simulink model "f0"  (Simulink 8.0) (continued)
== system f0/FIR/FIR_prog == (continued)
      mask help: A delay block that uses BRAM for its storage.
      mask init:
      | delay_bram_init(gcb, ...
      |     'DelayLen', DelayLen, ...
      |     'bram_latency', bram_latency, ...
      |     'use_dsp48', use_dsp48, ...
      |     'async', async);
      mask Help: eval('xlWeb(''http://casper.berkeley.edu/wiki/Delay_bram'')')
  delay6 : Subsystem  AncestorBlock=casper_library_delays/delay_bram  mask(delay_bram){DelayLen=256, bram_latency=2, use_dsp48=off, async=off}
      mask prompts: DelayLen "Delay By:"; bram_latency "BRAM Latency"; use_dsp48 "Use DSP48"; async "asynchronous operation"
      mask help: A delay block that uses BRAM for its storage.
      mask init:
      | delay_bram_init(gcb, ...
      |     'DelayLen', DelayLen, ...
      |     'bram_latency', bram_latency, ...
      |     'use_dsp48', use_dsp48, ...
      |     'async', async);
      mask Help: eval('xlWeb(''http://casper.berkeley.edu/wiki/Delay_bram'')')
  delay60 : Subsystem  AncestorBlock=casper_library_delays/delay_bram  mask(delay_bram){DelayLen=256, bram_latency=2, use_dsp48=off, async=off}
      mask prompts: DelayLen "Delay By:"; bram_latency "BRAM Latency"; use_dsp48 "Use DSP48"; async "asynchronous operation"
      mask help: A delay block that uses BRAM for its storage.
      mask init:
      | delay_bram_init(gcb, ...
      |     'DelayLen', DelayLen, ...
      |     'bram_latency', bram_latency, ...
      |     'use_dsp48', use_dsp48, ...
      |     'async', async);
      mask Help: eval('xlWeb(''http://casper.berkeley.edu/wiki/Delay_bram'')')
  delay61 : Subsystem  AncestorBlock=casper_library_delays/delay_bram  mask(delay_bram){DelayLen=256, bram_latency=2, use_dsp48=off, async=off}
      mask prompts: DelayLen "Delay By:"; bram_latency "BRAM Latency"; use_dsp48 "Use DSP48"; async "asynchronous operation"
      mask help: A delay block that uses BRAM for its storage.
      mask init:
      | delay_bram_init(gcb, ...
      |     'DelayLen', DelayLen, ...
      |     'bram_latency', bram_latency, ...
      |     'use_dsp48', use_dsp48, ...
      |     'async', async);
      mask Help: eval('xlWeb(''http://casper.berkeley.edu/wiki/Delay_bram'')')
  delay62 : Subsystem  AncestorBlock=casper_library_delays/delay_bram  mask(delay_bram){DelayLen=256, bram_latency=2, use_dsp48=off, async=off}
      mask prompts: DelayLen "Delay By:"; bram_latency "BRAM Latency"; use_dsp48 "Use DSP48"; async "asynchronous operation"
      mask help: A delay block that uses BRAM for its storage.
      mask init:
      | delay_bram_init(gcb, ...
      |     'DelayLen', DelayLen, ...
      |     'bram_latency', bram_latency, ...
      |     'use_dsp48', use_dsp48, ...
      |     'async', async);
      mask Help: eval('xlWeb(''http://casper.berkeley.edu/wiki/Delay_bram'')')
  delay63 : Subsystem  AncestorBlock=casper_library_delays/delay_bram  mask(delay_bram){DelayLen=256, bram_latency=2, use_dsp48=off, async=off}
      mask prompts: DelayLen "Delay By:"; bram_latency "BRAM Latency"; use_dsp48 "Use DSP48"; async "asynchronous operation"
      mask help: A delay block that uses BRAM for its storage.
      mask init:
      | delay_bram_init(gcb, ...
      |     'DelayLen', DelayLen, ...
      |     'bram_latency', bram_latency, ...
      |     'use_dsp48', use_dsp48, ...
      |     'async', async);
      mask Help: eval('xlWeb(''http://casper.berkeley.edu/wiki/Delay_bram'')')
  delay64 : Subsystem  AncestorBlock=casper_library_delays/delay_bram  mask(delay_bram){DelayLen=256, bram_latency=2, use_dsp48=off, async=off}
      mask prompts: DelayLen "Delay By:"; bram_latency "BRAM Latency"; use_dsp48 "Use DSP48"; async "asynchronous operation"
      mask help: A delay block that uses BRAM for its storage.
      mask init:
      | delay_bram_init(gcb, ...
      |     'DelayLen', DelayLen, ...
      |     'bram_latency', bram_latency, ...
      |     'use_dsp48', use_dsp48, ...
      |     'async', async);
      mask Help: eval('xlWeb(''http://casper.berkeley.edu/wiki/Delay_bram'')')
  delay65 : Subsystem  AncestorBlock=casper_library_delays/delay_bram  mask(delay_bram){DelayLen=256, bram_latency=2, use_dsp48=off, async=off}
      mask prompts: DelayLen "Delay By:"; bram_latency "BRAM Latency"; use_dsp48 "Use DSP48"; async "asynchronous operation"
      mask help: A delay block that uses BRAM for its storage.
      mask init:
      | delay_bram_init(gcb, ...
      |     'DelayLen', DelayLen, ...
      |     'bram_latency', bram_latency, ...
      |     'use_dsp48', use_dsp48, ...
      |     'async', async);
      mask Help: eval('xlWeb(''http://casper.berkeley.edu/wiki/Delay_bram'')')
  delay66 : Subsystem  AncestorBlock=casper_library_delays/delay_bram  mask(delay_bram){DelayLen=256, bram_latency=2, use_dsp48=off, async=off}
      mask prompts: DelayLen "Delay By:"; bram_latency "BRAM Latency"; use_dsp48 "Use DSP48"; async "asynchronous operation"
      mask help: A delay block that uses BRAM for its storage.
      mask init:
      | delay_bram_init(gcb, ...
      |     'DelayLen', DelayLen, ...
      |     'bram_latency', bram_latency, ...
      |     'use_dsp48', use_dsp48, ...
      |     'async', async);
      mask Help: eval('xlWeb(''http://casper.berkeley.edu/wiki/Delay_bram'')')
  delay67 : Subsystem  AncestorBlock=casper_library_delays/delay_bram  mask(delay_bram){DelayLen=256, bram_latency=2, use_dsp48=off, async=off}
      mask prompts: DelayLen "Delay By:"; bram_latency "BRAM Latency"; use_dsp48 "Use DSP48"; async "asynchronous operation"
      mask help: A delay block that uses BRAM for its storage.
      mask init:
      | delay_bram_init(gcb, ...
      |     'DelayLen', DelayLen, ...
      |     'bram_latency', bram_latency, ...
      |     'use_dsp48', use_dsp48, ...
      |     'async', async);
      mask Help: eval('xlWeb(''http://casper.berkeley.edu/wiki/Delay_bram'')')
  delay68 : Subsystem  AncestorBlock=casper_library_delays/delay_bram  mask(delay_bram){DelayLen=256, bram_latency=2, use_dsp48=off, async=off}
      mask prompts: DelayLen "Delay By:"; bram_latency "BRAM Latency"; use_dsp48 "Use DSP48"; async "asynchronous operation"
      mask help: A delay block that uses BRAM for its storage.
      mask init:
      | delay_bram_init(gcb, ...
      |     'DelayLen', DelayLen, ...
      |     'bram_latency', bram_latency, ...
      |     'use_dsp48', use_dsp48, ...
      |     'async', async);
      mask Help: eval('xlWeb(''http://casper.berkeley.edu/wiki/Delay_bram'')')
  delay69 : Subsystem  AncestorBlock=casper_library_delays/delay_bram  mask(delay_bram){DelayLen=256, bram_latency=2, use_dsp48=off, async=off}
      mask prompts: DelayLen "Delay By:"; bram_latency "BRAM Latency"; use_dsp48 "Use DSP48"; async "asynchronous operation"
      mask help: A delay block that uses BRAM for its storage.
      mask init:
      | delay_bram_init(gcb, ...
      |     'DelayLen', DelayLen, ...
      |     'bram_latency', bram_latency, ...
      |     'use_dsp48', use_dsp48, ...
      |     'async', async);
      mask Help: eval('xlWeb(''http://casper.berkeley.edu/wiki/Delay_bram'')')
  delay7 : Subsystem  AncestorBlock=casper_library_delays/delay_bram  mask(delay_bram){DelayLen=256, bram_latency=2, use_dsp48=off, async=off}
      mask prompts: DelayLen "Delay By:"; bram_latency "BRAM Latency"; use_dsp48 "Use DSP48"; async "asynchronous operation"
      mask help: A delay block that uses BRAM for its storage.
      mask init:
      | delay_bram_init(gcb, ...
      |     'DelayLen', DelayLen, ...
      |     'bram_latency', bram_latency, ...
      |     'use_dsp48', use_dsp48, ...
      |     'async', async);
      mask Help: eval('xlWeb(''http://casper.berkeley.edu/wiki/Delay_bram'')')
  delay70 : Subsystem  AncestorBlock=casper_library_delays/delay_bram  mask(delay_bram){DelayLen=256, bram_latency=2, use_dsp48=off, async=off}
      mask prompts: DelayLen "Delay By:"; bram_latency "BRAM Latency"; use_dsp48 "Use DSP48"; async "asynchronous operation"
      mask help: A delay block that uses BRAM for its storage.
      mask init:
      | delay_bram_init(gcb, ...
      |     'DelayLen', DelayLen, ...
      |     'bram_latency', bram_latency, ...
      |     'use_dsp48', use_dsp48, ...
      |     'async', async);
      mask Help: eval('xlWeb(''http://casper.berkeley.edu/wiki/Delay_bram'')')
  delay71 : Subsystem  AncestorBlock=casper_library_delays/delay_bram  mask(delay_bram){DelayLen=256, bram_latency=2, use_dsp48=off, async=off}
      mask prompts: DelayLen "Delay By:"; bram_latency "BRAM Latency"; use_dsp48 "Use DSP48"; async "asynchronous operation"
      mask help: A delay block that uses BRAM for its storage.
      mask init:
      | delay_bram_init(gcb, ...
      |     'DelayLen', DelayLen, ...
      |     'bram_latency', bram_latency, ...
      |     'use_dsp48', use_dsp48, ...
      |     'async', async);
      mask Help: eval('xlWeb(''http://casper.berkeley.edu/wiki/Delay_bram'')')
  delay72 : Subsystem  AncestorBlock=casper_library_delays/delay_bram  mask(delay_bram){DelayLen=256, bram_latency=2, use_dsp48=off, async=off}
      mask prompts: DelayLen "Delay By:"; bram_latency "BRAM Latency"; use_dsp48 "Use DSP48"; async "asynchronous operation"
      mask help: A delay block that uses BRAM for its storage.
      mask init:
      | delay_bram_init(gcb, ...
      |     'DelayLen', DelayLen, ...
      |     'bram_latency', bram_latency, ...
      |     'use_dsp48', use_dsp48, ...
      |     'async', async);
      mask Help: eval('xlWeb(''http://casper.berkeley.edu/wiki/Delay_bram'')')
  delay73 : Subsystem  AncestorBlock=casper_library_delays/delay_bram  mask(delay_bram){DelayLen=256, bram_latency=2, use_dsp48=off, async=off}
      mask prompts: DelayLen "Delay By:"; bram_latency "BRAM Latency"; use_dsp48 "Use DSP48"; async "asynchronous operation"
      mask help: A delay block that uses BRAM for its storage.
      mask init:
      | delay_bram_init(gcb, ...
      |     'DelayLen', DelayLen, ...
      |     'bram_latency', bram_latency, ...
      |     'use_dsp48', use_dsp48, ...
      |     'async', async);
      mask Help: eval('xlWeb(''http://casper.berkeley.edu/wiki/Delay_bram'')')
  delay74 : Subsystem  AncestorBlock=casper_library_delays/delay_bram  mask(delay_bram){DelayLen=256, bram_latency=2, use_dsp48=off, async=off}
      mask prompts: DelayLen "Delay By:"; bram_latency "BRAM Latency"; use_dsp48 "Use DSP48"; async "asynchronous operation"
      mask help: A delay block that uses BRAM for its storage.
      mask init:
      | delay_bram_init(gcb, ...
      |     'DelayLen', DelayLen, ...
      |     'bram_latency', bram_latency, ...
      |     'use_dsp48', use_dsp48, ...
      |     'async', async);
      mask Help: eval('xlWeb(''http://casper.berkeley.edu/wiki/Delay_bram'')')
  delay75 : Subsystem  AncestorBlock=casper_library_delays/delay_bram  mask(delay_bram){DelayLen=256, bram_latency=2, use_dsp48=off, async=off}
      mask prompts: DelayLen "Delay By:"; bram_latency "BRAM Latency"; use_dsp48 "Use DSP48"; async "asynchronous operation"
      mask help: A delay block that uses BRAM for its storage.
      mask init:
      | delay_bram_init(gcb, ...
      |     'DelayLen', DelayLen, ...
      |     'bram_latency', bram_latency, ...
      |     'use_dsp48', use_dsp48, ...
      |     'async', async);
      mask Help: eval('xlWeb(''http://casper.berkeley.edu/wiki/Delay_bram'')')
  delay76 : Subsystem  AncestorBlock=casper_library_delays/delay_bram  mask(delay_bram){DelayLen=256, bram_latency=2, use_dsp48=off, async=off}
      mask prompts: DelayLen "Delay By:"; bram_latency "BRAM Latency"; use_dsp48 "Use DSP48"; async "asynchronous operation"
      mask help: A delay block that uses BRAM for its storage.
      mask init:
      | delay_bram_init(gcb, ...
      |     'DelayLen', DelayLen, ...
      |     'bram_latency', bram_latency, ...
      |     'use_dsp48', use_dsp48, ...
      |     'async', async);
      mask Help: eval('xlWeb(''http://casper.berkeley.edu/wiki/Delay_bram'')')
  delay77 : Subsystem  AncestorBlock=casper_library_delays/delay_bram  mask(delay_bram){DelayLen=256, bram_latency=2, use_dsp48=off, async=off}
      mask prompts: DelayLen "Delay By:"; bram_latency "BRAM Latency"; use_dsp48 "Use DSP48"; async "asynchronous operation"
      mask help: A delay block that uses BRAM for its storage.
      mask init:
      | delay_bram_init(gcb, ...
      |     'DelayLen', DelayLen, ...
      |     'bram_latency', bram_latency, ...
      |     'use_dsp48', use_dsp48, ...
      |     'async', async);
      mask Help: eval('xlWeb(''http://casper.berkeley.edu/wiki/Delay_bram'')')
  delay78 : Subsystem  AncestorBlock=casper_library_delays/delay_bram  mask(delay_bram){DelayLen=256, bram_latency=2, use_dsp48=off, async=off}
      mask prompts: DelayLen "Delay By:"; bram_latency "BRAM Latency"; use_dsp48 "Use DSP48"; async "asynchronous operation"
      mask help: A delay block that uses BRAM for its storage.
      mask init:
      | delay_bram_init(gcb, ...
      |     'DelayLen', DelayLen, ...
      |     'bram_latency', bram_latency, ...
      |     'use_dsp48', use_dsp48, ...
      |     'async', async);
      mask Help: eval('xlWeb(''http://casper.berkeley.edu/wiki/Delay_bram'')')
  delay79 : Subsystem  AncestorBlock=casper_library_delays/delay_bram  mask(delay_bram){DelayLen=256, bram_latency=2, use_dsp48=off, async=off}
      mask prompts: DelayLen "Delay By:"; bram_latency "BRAM Latency"; use_dsp48 "Use DSP48"; async "asynchronous operation"
      mask help: A delay block that uses BRAM for its storage.
      mask init:
      | delay_bram_init(gcb, ...
      |     'DelayLen', DelayLen, ...
      |     'bram_latency', bram_latency, ...
      |     'use_dsp48', use_dsp48, ...
      |     'async', async);
      mask Help: eval('xlWeb(''http://casper.berkeley.edu/wiki/Delay_bram'')')
  delay8 : Subsystem  AncestorBlock=casper_library_delays/delay_bram  mask(delay_bram){DelayLen=256, bram_latency=2, use_dsp48=off, async=off}
      mask prompts: DelayLen "Delay By:"; bram_latency "BRAM Latency"; use_dsp48 "Use DSP48"; async "asynchronous operation"
      mask help: A delay block that uses BRAM for its storage.
      mask init:
      | delay_bram_init(gcb, ...
      |     'DelayLen', DelayLen, ...
      |     'bram_latency', bram_latency, ...
      |     'use_dsp48', use_dsp48, ...
      |     'async', async);
      mask Help: eval('xlWeb(''http://casper.berkeley.edu/wiki/Delay_bram'')')
  delay80 : Subsystem  AncestorBlock=casper_library_delays/delay_bram  mask(delay_bram){DelayLen=256, bram_latency=2, use_dsp48=off, async=off}
      mask prompts: DelayLen "Delay By:"; bram_latency "BRAM Latency"; use_dsp48 "Use DSP48"; async "asynchronous operation"
      mask help: A delay block that uses BRAM for its storage.
      mask init:
      | delay_bram_init(gcb, ...
      |     'DelayLen', DelayLen, ...
      |     'bram_latency', bram_latency, ...
      |     'use_dsp48', use_dsp48, ...
      |     'async', async);
      mask Help: eval('xlWeb(''http://casper.berkeley.edu/wiki/Delay_bram'')')
  delay81 : Subsystem  AncestorBlock=casper_library_delays/delay_bram  mask(delay_bram){DelayLen=256, bram_latency=2, use_dsp48=off, async=off}
      mask prompts: DelayLen "Delay By:"; bram_latency "BRAM Latency"; use_dsp48 "Use DSP48"; async "asynchronous operation"
      mask help: A delay block that uses BRAM for its storage.
      mask init:
      | delay_bram_init(gcb, ...
      |     'DelayLen', DelayLen, ...
      |     'bram_latency', bram_latency, ...
      |     'use_dsp48', use_dsp48, ...
      |     'async', async);
      mask Help: eval('xlWeb(''http://casper.berkeley.edu/wiki/Delay_bram'')')
  delay82 : Subsystem  AncestorBlock=casper_library_delays/delay_bram  mask(delay_bram){DelayLen=256, bram_latency=2, use_dsp48=off, async=off}
      mask prompts: DelayLen "Delay By:"; bram_latency "BRAM Latency"; use_dsp48 "Use DSP48"; async "asynchronous operation"
      mask help: A delay block that uses BRAM for its storage.
      mask init:
      | delay_bram_init(gcb, ...
      |     'DelayLen', DelayLen, ...
      |     'bram_latency', bram_latency, ...
      |     'use_dsp48', use_dsp48, ...
      |     'async', async);
      mask Help: eval('xlWeb(''http://casper.berkeley.edu/wiki/Delay_bram'')')
  delay83 : Subsystem  AncestorBlock=casper_library_delays/delay_bram  mask(delay_bram){DelayLen=256, bram_latency=2, use_dsp48=off, async=off}
      mask prompts: DelayLen "Delay By:"; bram_latency "BRAM Latency"; use_dsp48 "Use DSP48"; async "asynchronous operation"
      mask help: A delay block that uses BRAM for its storage.
      mask init:
      | delay_bram_init(gcb, ...
      |     'DelayLen', DelayLen, ...
      |     'bram_latency', bram_latency, ...
      |     'use_dsp48', use_dsp48, ...
      |     'async', async);
      mask Help: eval('xlWeb(''http://casper.berkeley.edu/wiki/Delay_bram'')')
  delay84 : Subsystem  AncestorBlock=casper_library_delays/delay_bram  mask(delay_bram){DelayLen=256, bram_latency=2, use_dsp48=off, async=off}
      mask prompts: DelayLen "Delay By:"; bram_latency "BRAM Latency"; use_dsp48 "Use DSP48"; async "asynchronous operation"
      mask help: A delay block that uses BRAM for its storage.
      mask init:
      | delay_bram_init(gcb, ...
      |     'DelayLen', DelayLen, ...
      |     'bram_latency', bram_latency, ...
      |     'use_dsp48', use_dsp48, ...
      |     'async', async);
      mask Help: eval('xlWeb(''http://casper.berkeley.edu/wiki/Delay_bram'')')
  delay85 : Subsystem  AncestorBlock=casper_library_delays/delay_bram  mask(delay_bram){DelayLen=256, bram_latency=2, use_dsp48=off, async=off}
      mask prompts: DelayLen "Delay By:"; bram_latency "BRAM Latency"; use_dsp48 "Use DSP48"; async "asynchronous operation"
      mask help: A delay block that uses BRAM for its storage.
      mask init:
      | delay_bram_init(gcb, ...
      |     'DelayLen', DelayLen, ...
      |     'bram_latency', bram_latency, ...
      |     'use_dsp48', use_dsp48, ...
      |     'async', async);
      mask Help: eval('xlWeb(''http://casper.berkeley.edu/wiki/Delay_bram'')')
  delay86 : Subsystem  AncestorBlock=casper_library_delays/delay_bram  mask(delay_bram){DelayLen=256, bram_latency=2, use_dsp48=off, async=off}
      mask prompts: DelayLen "Delay By:"; bram_latency "BRAM Latency"; use_dsp48 "Use DSP48"; async "asynchronous operation"
      mask help: A delay block that uses BRAM for its storage.
      mask init:
      | delay_bram_init(gcb, ...
      |     'DelayLen', DelayLen, ...
      |     'bram_latency', bram_latency, ...
      |     'use_dsp48', use_dsp48, ...
      |     'async', async);
      mask Help: eval('xlWeb(''http://casper.berkeley.edu/wiki/Delay_bram'')')
  delay87 : Subsystem  AncestorBlock=casper_library_delays/delay_bram  mask(delay_bram){DelayLen=256, bram_latency=2, use_dsp48=off, async=off}
      mask prompts: DelayLen "Delay By:"; bram_latency "BRAM Latency"; use_dsp48 "Use DSP48"; async "asynchronous operation"
      mask help: A delay block that uses BRAM for its storage.
      mask init:
      | delay_bram_init(gcb, ...
      |     'DelayLen', DelayLen, ...
      |     'bram_latency', bram_latency, ...
      |     'use_dsp48', use_dsp48, ...
      |     'async', async);
      mask Help: eval('xlWeb(''http://casper.berkeley.edu/wiki/Delay_bram'')')
  delay88 : Subsystem  AncestorBlock=casper_library_delays/delay_bram  mask(delay_bram){DelayLen=256, bram_latency=2, use_dsp48=off, async=off}
      mask prompts: DelayLen "Delay By:"; bram_latency "BRAM Latency"; use_dsp48 "Use DSP48"; async "asynchronous operation"
      mask help: A delay block that uses BRAM for its storage.
      mask init:
      | delay_bram_init(gcb, ...
      |     'DelayLen', DelayLen, ...
      |     'bram_latency', bram_latency, ...
      |     'use_dsp48', use_dsp48, ...
      |     'async', async);
      mask Help: eval('xlWeb(''http://casper.berkeley.edu/wiki/Delay_bram'')')
  delay89 : Subsystem  AncestorBlock=casper_library_delays/delay_bram  mask(delay_bram){DelayLen=256, bram_latency=2, use_dsp48=off, async=off}
      mask prompts: DelayLen "Delay By:"; bram_latency "BRAM Latency"; use_dsp48 "Use DSP48"; async "asynchronous operation"
      mask help: A delay block that uses BRAM for its storage.
      mask init:
      | delay_bram_init(gcb, ...
      |     'DelayLen', DelayLen, ...
      |     'bram_latency', bram_latency, ...
      |     'use_dsp48', use_dsp48, ...
      |     'async', async);
      mask Help: eval('xlWeb(''http://casper.berkeley.edu/wiki/Delay_bram'')')
  delay9 : Subsystem  AncestorBlock=casper_library_delays/delay_bram  mask(delay_bram){DelayLen=256, bram_latency=2, use_dsp48=off, async=off}
      mask prompts: DelayLen "Delay By:"; bram_latency "BRAM Latency"; use_dsp48 "Use DSP48"; async "asynchronous operation"
      mask help: A delay block that uses BRAM for its storage.
      mask init:
      | delay_bram_init(gcb, ...
      |     'DelayLen', DelayLen, ...
      |     'bram_latency', bram_latency, ...
      |     'use_dsp48', use_dsp48, ...
      |     'async', async);
      mask Help: eval('xlWeb(''http://casper.berkeley.edu/wiki/Delay_bram'')')
  delay90 : Subsystem  AncestorBlock=casper_library_delays/delay_bram  mask(delay_bram){DelayLen=256, bram_latency=2, use_dsp48=off, async=off}
      mask prompts: DelayLen "Delay By:"; bram_latency "BRAM Latency"; use_dsp48 "Use DSP48"; async "asynchronous operation"
      mask help: A delay block that uses BRAM for its storage.
      mask init:
      | delay_bram_init(gcb, ...
      |     'DelayLen', DelayLen, ...
      |     'bram_latency', bram_latency, ...
      |     'use_dsp48', use_dsp48, ...
      |     'async', async);
      mask Help: eval('xlWeb(''http://casper.berkeley.edu/wiki/Delay_bram'')')
  delay91 : Subsystem  AncestorBlock=casper_library_delays/delay_bram  mask(delay_bram){DelayLen=256, bram_latency=2, use_dsp48=off, async=off}
      mask prompts: DelayLen "Delay By:"; bram_latency "BRAM Latency"; use_dsp48 "Use DSP48"; async "asynchronous operation"
      mask help: A delay block that uses BRAM for its storage.
      mask init:
      | delay_bram_init(gcb, ...
      |     'DelayLen', DelayLen, ...
      |     'bram_latency', bram_latency, ...
      |     'use_dsp48', use_dsp48, ...
      |     'async', async);
      mask Help: eval('xlWeb(''http://casper.berkeley.edu/wiki/Delay_bram'')')
  delay92 : Subsystem  AncestorBlock=casper_library_delays/delay_bram  mask(delay_bram){DelayLen=256, bram_latency=2, use_dsp48=off, async=off}
      mask prompts: DelayLen "Delay By:"; bram_latency "BRAM Latency"; use_dsp48 "Use DSP48"; async "asynchronous operation"
      mask help: A delay block that uses BRAM for its storage.
      mask init:
      | delay_bram_init(gcb, ...
      |     'DelayLen', DelayLen, ...
      |     'bram_latency', bram_latency, ...
      |     'use_dsp48', use_dsp48, ...
      |     'async', async);
      mask Help: eval('xlWeb(''http://casper.berkeley.edu/wiki/Delay_bram'')')
  delay93 : Subsystem  AncestorBlock=casper_library_delays/delay_bram  mask(delay_bram){DelayLen=256, bram_latency=2, use_dsp48=off, async=off}
      mask prompts: DelayLen "Delay By:"; bram_latency "BRAM Latency"; use_dsp48 "Use DSP48"; async "asynchronous operation"
      mask help: A delay block that uses BRAM for its storage.
      mask init:
      | delay_bram_init(gcb, ...
      |     'DelayLen', DelayLen, ...
      |     'bram_latency', bram_latency, ...
      |     'use_dsp48', use_dsp48, ...
      |     'async', async);
      mask Help: eval('xlWeb(''http://casper.berkeley.edu/wiki/Delay_bram'')')
  delay94 : Subsystem  AncestorBlock=casper_library_delays/delay_bram  mask(delay_bram){DelayLen=256, bram_latency=2, use_dsp48=off, async=off}
      mask prompts: DelayLen "Delay By:"; bram_latency "BRAM Latency"; use_dsp48 "Use DSP48"; async "asynchronous operation"
      mask help: A delay block that uses BRAM for its storage.
      mask init:
      | delay_bram_init(gcb, ...
      |     'DelayLen', DelayLen, ...
      |     'bram_latency', bram_latency, ...
      |     'use_dsp48', use_dsp48, ...
      |     'async', async);
      mask Help: eval('xlWeb(''http://casper.berkeley.edu/wiki/Delay_bram'')')
  delay95 : Subsystem  AncestorBlock=casper_library_delays/delay_bram  mask(delay_bram){DelayLen=256, bram_latency=2, use_dsp48=off, async=off}
      mask prompts: DelayLen "Delay By:"; bram_latency "BRAM Latency"; use_dsp48 "Use DSP48"; async "asynchronous operation"
      mask help: A delay block that uses BRAM for its storage.
      mask init:
      | delay_bram_init(gcb, ...
      |     'DelayLen', DelayLen, ...
      |     'bram_latency', bram_latency, ...
      |     'use_dsp48', use_dsp48, ...
      |     'async', async);
      mask Help: eval('xlWeb(''http://casper.berkeley.edu/wiki/Delay_bram'')')
  delay96 : Subsystem  AncestorBlock=casper_library_delays/delay_bram  mask(delay_bram){DelayLen=256, bram_latency=2, use_dsp48=off, async=off}
      mask prompts: DelayLen "Delay By:"; bram_latency "BRAM Latency"; use_dsp48 "Use DSP48"; async "asynchronous operation"
      mask help: A delay block that uses BRAM for its storage.
      mask init:
      | delay_bram_init(gcb, ...
      |     'DelayLen', DelayLen, ...
      |     'bram_latency', bram_latency, ...
      |     'use_dsp48', use_dsp48, ...
      |     'async', async);
      mask Help: eval('xlWeb(''http://casper.berkeley.edu/wiki/Delay_bram'')')
  delay97 : Subsystem  AncestorBlock=casper_library_delays/delay_bram  mask(delay_bram){DelayLen=256, bram_latency=2, use_dsp48=off, async=off}
      mask prompts: DelayLen "Delay By:"; bram_latency "BRAM Latency"; use_dsp48 "Use DSP48"; async "asynchronous operation"
      mask help: A delay block that uses BRAM for its storage.
      mask init:
      | delay_bram_init(gcb, ...
      |     'DelayLen', DelayLen, ...
      |     'bram_latency', bram_latency, ...
      |     'use_dsp48', use_dsp48, ...
      |     'async', async);
      mask Help: eval('xlWeb(''http://casper.berkeley.edu/wiki/Delay_bram'')')
  delay98 : Subsystem  AncestorBlock=casper_library_delays/delay_bram  mask(delay_bram){DelayLen=256, bram_latency=2, use_dsp48=off, async=off}
      mask prompts: DelayLen "Delay By:"; bram_latency "BRAM Latency"; use_dsp48 "Use DSP48"; async "asynchronous operation"
      mask help: A delay block that uses BRAM for its storage.
      mask init:
      | delay_bram_init(gcb, ...
      |     'DelayLen', DelayLen, ...
      |     'bram_latency', bram_latency, ...
      |     'use_dsp48', use_dsp48, ...
      |     'async', async);
      mask Help: eval('xlWeb(''http://casper.berkeley.edu/wiki/Delay_bram'')')
  delay99 : Subsystem  AncestorBlock=casper_library_delays/delay_bram  mask(delay_bram){DelayLen=256, bram_latency=2, use_dsp48=off, async=off}
      mask prompts: DelayLen "Delay By:"; bram_latency "BRAM Latency"; use_dsp48 "Use DSP48"; async "asynchronous operation"
      mask help: A delay block that uses BRAM for its storage.
      mask init:
      | delay_bram_init(gcb, ...
      |     'DelayLen', DelayLen, ...
      |     'bram_latency', bram_latency, ...
      |     'use_dsp48', use_dsp48, ...
      |     'async', async);
      mask Help: eval('xlWeb(''http://casper.berkeley.edu/wiki/Delay_bram'')')
  sum : Subsystem  AncestorBlock=casper_library_misc/adder_tree  mask(adder_tree){n_inputs=100, latency=3, adder_imp=DSP48, first_stage_hdl=on}
      mask prompts: n_inputs "Number of Inputs"; latency "Add Latency"; adder_imp "Adder implementation"; first_stage_hdl "Use behavioural HDL for first stage of adders"
      mask help:
      | Sums all inputs using a tree of adds and delays.
      |
      | Number of inputs: The number of inputs to the block to be summed
      | Add latency: The number of clock cycles to perform one addition (affects timing)..
      | Adder implementation: choice of fabric or DSP48E core, or behavioral HDL
      | Use behavioural HDL for first stage of adders: Causes the first stage of adders to be absorbed into DSP blocks if multipliers precede them (Virtex 5). Overrides Adder implementation
      mask init:
      | adder_tree_init(gcb, ...
      |     'n_inputs', n_inputs, ...
      |     'latency', latency, ...
      |     'adder_imp', adder_imp, ...
      |     'first_stage_hdl', first_stage_hdl);
      settings: AttributesFormatString=latency 21
      mask Help: eval('xlWeb(''http://casper.berkeley.edu/wiki/Adder_tree'')')
      mask TypeOptions adder_imp: Behavioral | Fabric | DSP48
  sync_delay : xbsIndex_r4/Delay  rst=off  infoeditControl=Selection of Reset will increase slice count due to use of real FFs and instead of SRLs  en=off  latency=5  dbl_ovrd=off  reg_retiming=off  block_type=delay
  sync_out : Outport
  data_out : Outport  Port=2
  wires:
  sync_in/1 -> sync_delay/1
  sum/2(dout) -> data_out/1
  sync_delay/1 -> sum/1(sync)
  sum/1(sync_out) -> sync_out/1
  coeff1/1 -> Mult1/2
  Mult1/1 -> sum/2(din1)
  coeff2/1 -> Mult2/2
  delay1/1(dout) -> Mult2/1, delay2/1(din)
  Mult2/1 -> sum/3(din2)
  coeff3/1 -> Mult3/2
  delay2/1(dout) -> Mult3/1, delay3/1(din)
  Mult3/1 -> sum/4(din3)
  coeff4/1 -> Mult4/2
  delay3/1(dout) -> Mult4/1, delay4/1(din)
  Mult4/1 -> sum/5(din4)
  coeff5/1 -> Mult5/2
  delay4/1(dout) -> Mult5/1, delay5/1(din)
  Mult5/1 -> sum/6(din5)
  coeff6/1 -> Mult6/2
  delay5/1(dout) -> Mult6/1, delay6/1(din)
  Mult6/1 -> sum/7(din6)
  coeff7/1 -> Mult7/2
  delay6/1(dout) -> Mult7/1, delay7/1(din)
  Mult7/1 -> sum/8(din7)
  coeff8/1 -> Mult8/2
  delay7/1(dout) -> Mult8/1, delay8/1(din)
  Mult8/1 -> sum/9(din8)
  coeff9/1 -> Mult9/2
  delay8/1(dout) -> Mult9/1, delay9/1(din)
  Mult9/1 -> sum/10(din9)
  coeff10/1 -> Mult10/2
  delay9/1(dout) -> Mult10/1, delay10/1(din)
  Mult10/1 -> sum/11(din10)
  coeff11/1 -> Mult11/2
  delay10/1(dout) -> Mult11/1, delay11/1(din)
  Mult11/1 -> sum/12(din11)
  coeff12/1 -> Mult12/2
  delay11/1(dout) -> Mult12/1, delay12/1(din)
  Mult12/1 -> sum/13(din12)
  coeff13/1 -> Mult13/2
  delay12/1(dout) -> Mult13/1, delay13/1(din)
  Mult13/1 -> sum/14(din13)
  coeff14/1 -> Mult14/2
  delay13/1(dout) -> Mult14/1, delay14/1(din)
  Mult14/1 -> sum/15(din14)
  coeff15/1 -> Mult15/2
  delay14/1(dout) -> Mult15/1, delay15/1(din)
  Mult15/1 -> sum/16(din15)
  coeff16/1 -> Mult16/2
  delay15/1(dout) -> Mult16/1, delay16/1(din)
  Mult16/1 -> sum/17(din16)
  coeff17/1 -> Mult17/2
  delay16/1(dout) -> Mult17/1, delay17/1(din)
  Mult17/1 -> sum/18(din17)
  coeff18/1 -> Mult18/2
  delay17/1(dout) -> Mult18/1, delay18/1(din)
  Mult18/1 -> sum/19(din18)
  coeff19/1 -> Mult19/2
  delay18/1(dout) -> Mult19/1, delay19/1(din)
  Mult19/1 -> sum/20(din19)
  coeff20/1 -> Mult20/2
  delay19/1(dout) -> Mult20/1, delay20/1(din)
  Mult20/1 -> sum/21(din20)
  coeff21/1 -> Mult21/2
  delay20/1(dout) -> Mult21/1, delay21/1(din)
  Mult21/1 -> sum/22(din21)
  coeff22/1 -> Mult22/2
  delay21/1(dout) -> Mult22/1, delay22/1(din)
  Mult22/1 -> sum/23(din22)
  coeff23/1 -> Mult23/2
  delay22/1(dout) -> Mult23/1, delay23/1(din)
  Mult23/1 -> sum/24(din23)
  coeff24/1 -> Mult24/2
  delay23/1(dout) -> Mult24/1, delay24/1(din)
  Mult24/1 -> sum/25(din24)
  coeff25/1 -> Mult25/2
  delay24/1(dout) -> Mult25/1, delay25/1(din)
  Mult25/1 -> sum/26(din25)
  coeff26/1 -> Mult26/2
  delay25/1(dout) -> Mult26/1, delay26/1(din)
  Mult26/1 -> sum/27(din26)
  coeff27/1 -> Mult27/2
  delay26/1(dout) -> Mult27/1, delay27/1(din)
  Mult27/1 -> sum/28(din27)
  coeff28/1 -> Mult28/2
  delay27/1(dout) -> Mult28/1, delay28/1(din)
  Mult28/1 -> sum/29(din28)
  coeff29/1 -> Mult29/2
  delay28/1(dout) -> Mult29/1, delay29/1(din)
  Mult29/1 -> sum/30(din29)
  coeff30/1 -> Mult30/2
  delay29/1(dout) -> Mult30/1, delay30/1(din)
  Mult30/1 -> sum/31(din30)
  coeff31/1 -> Mult31/2
  delay30/1(dout) -> Mult31/1, delay31/1(din)
  Mult31/1 -> sum/32(din31)
  coeff32/1 -> Mult32/2
  delay31/1(dout) -> Mult32/1, delay32/1(din)
  Mult32/1 -> sum/33(din32)
  coeff33/1 -> Mult33/2
  delay32/1(dout) -> Mult33/1, delay33/1(din)
  Mult33/1 -> sum/34(din33)
  coeff34/1 -> Mult34/2
  delay33/1(dout) -> Mult34/1, delay34/1(din)
  Mult34/1 -> sum/35(din34)
  coeff35/1 -> Mult35/2
  delay34/1(dout) -> Mult35/1, delay35/1(din)
  Mult35/1 -> sum/36(din35)
  coeff36/1 -> Mult36/2
  delay35/1(dout) -> Mult36/1, delay36/1(din)
  Mult36/1 -> sum/37(din36)
  coeff37/1 -> Mult37/2
  delay36/1(dout) -> Mult37/1, delay37/1(din)
  Mult37/1 -> sum/38(din37)
  coeff38/1 -> Mult38/2
  delay37/1(dout) -> Mult38/1, delay38/1(din)
  Mult38/1 -> sum/39(din38)
  coeff39/1 -> Mult39/2
  delay38/1(dout) -> Mult39/1, delay39/1(din)
  Mult39/1 -> sum/40(din39)
  coeff40/1 -> Mult40/2
  delay39/1(dout) -> Mult40/1, delay40/1(din)
  Mult40/1 -> sum/41(din40)
  coeff41/1 -> Mult41/2
  delay40/1(dout) -> Mult41/1, delay41/1(din)
  Mult41/1 -> sum/42(din41)
  coeff42/1 -> Mult42/2
  delay41/1(dout) -> Mult42/1, delay42/1(din)
  Mult42/1 -> sum/43(din42)
  coeff43/1 -> Mult43/2
  delay42/1(dout) -> Mult43/1, delay43/1(din)
  Mult43/1 -> sum/44(din43)
  coeff44/1 -> Mult44/2
  delay43/1(dout) -> Mult44/1, delay44/1(din)
  Mult44/1 -> sum/45(din44)
  coeff45/1 -> Mult45/2
  delay44/1(dout) -> Mult45/1, delay45/1(din)
  Mult45/1 -> sum/46(din45)
  coeff46/1 -> Mult46/2
  delay45/1(dout) -> Mult46/1, delay46/1(din)
  Mult46/1 -> sum/47(din46)
  coeff47/1 -> Mult47/2
  delay46/1(dout) -> Mult47/1, delay47/1(din)
  Mult47/1 -> sum/48(din47)
  coeff48/1 -> Mult48/2
  delay47/1(dout) -> Mult48/1, delay48/1(din)
  Mult48/1 -> sum/49(din48)
  coeff49/1 -> Mult49/2
  delay48/1(dout) -> Mult49/1, delay49/1(din)
  Mult49/1 -> sum/50(din49)
  coeff50/1 -> Mult50/2
  delay49/1(dout) -> Mult50/1, delay50/1(din)
  Mult50/1 -> sum/51(din50)
  coeff51/1 -> Mult51/2
  delay50/1(dout) -> Mult51/1, delay51/1(din)
  Mult51/1 -> sum/52(din51)
  coeff52/1 -> Mult52/2
  delay51/1(dout) -> Mult52/1, delay52/1(din)
  Mult52/1 -> sum/53(din52)
  coeff53/1 -> Mult53/2
  delay52/1(dout) -> Mult53/1, delay53/1(din)
  Mult53/1 -> sum/54(din53)
  coeff54/1 -> Mult54/2
  delay53/1(dout) -> Mult54/1, delay54/1(din)
  Mult54/1 -> sum/55(din54)
  coeff55/1 -> Mult55/2
  delay54/1(dout) -> Mult55/1, delay55/1(din)
  Mult55/1 -> sum/56(din55)
  coeff56/1 -> Mult56/2
  delay55/1(dout) -> Mult56/1, delay56/1(din)
  Mult56/1 -> sum/57(din56)
  coeff57/1 -> Mult57/2
  delay56/1(dout) -> Mult57/1, delay57/1(din)
  Mult57/1 -> sum/58(din57)
  coeff58/1 -> Mult58/2
  delay57/1(dout) -> Mult58/1, delay58/1(din)
  Mult58/1 -> sum/59(din58)
  coeff59/1 -> Mult59/2
  delay58/1(dout) -> Mult59/1, delay59/1(din)
  Mult59/1 -> sum/60(din59)
  coeff60/1 -> Mult60/2
  delay59/1(dout) -> Mult60/1, delay60/1(din)
  Mult60/1 -> sum/61(din60)
  coeff61/1 -> Mult61/2
  delay60/1(dout) -> Mult61/1, delay61/1(din)
  Mult61/1 -> sum/62(din61)
  coeff62/1 -> Mult62/2
  delay61/1(dout) -> Mult62/1, delay62/1(din)
  Mult62/1 -> sum/63(din62)
  coeff63/1 -> Mult63/2
  delay62/1(dout) -> Mult63/1, delay63/1(din)
  Mult63/1 -> sum/64(din63)
  coeff64/1 -> Mult64/2
  delay63/1(dout) -> Mult64/1, delay64/1(din)
  Mult64/1 -> sum/65(din64)
  coeff65/1 -> Mult65/2
  delay64/1(dout) -> Mult65/1, delay65/1(din)
  Mult65/1 -> sum/66(din65)
  coeff66/1 -> Mult66/2
  delay65/1(dout) -> Mult66/1, delay66/1(din)
  Mult66/1 -> sum/67(din66)
  coeff67/1 -> Mult67/2
  delay66/1(dout) -> Mult67/1, delay67/1(din)
  Mult67/1 -> sum/68(din67)
  coeff68/1 -> Mult68/2
  delay67/1(dout) -> Mult68/1, delay68/1(din)
  Mult68/1 -> sum/69(din68)
  coeff69/1 -> Mult69/2
  delay68/1(dout) -> Mult69/1, delay69/1(din)
  Mult69/1 -> sum/70(din69)
  coeff70/1 -> Mult70/2
  delay69/1(dout) -> Mult70/1, delay70/1(din)
  Mult70/1 -> sum/71(din70)
  coeff71/1 -> Mult71/2
  delay70/1(dout) -> Mult71/1, delay71/1(din)
  Mult71/1 -> sum/72(din71)
  coeff72/1 -> Mult72/2
  delay71/1(dout) -> Mult72/1, delay72/1(din)
  Mult72/1 -> sum/73(din72)
  coeff73/1 -> Mult73/2
  delay72/1(dout) -> Mult73/1, delay73/1(din)
  Mult73/1 -> sum/74(din73)
  coeff74/1 -> Mult74/2
  delay73/1(dout) -> Mult74/1, delay74/1(din)
  Mult74/1 -> sum/75(din74)
  coeff75/1 -> Mult75/2
  delay74/1(dout) -> Mult75/1, delay75/1(din)
  Mult75/1 -> sum/76(din75)
  coeff76/1 -> Mult76/2
  delay75/1(dout) -> Mult76/1, delay76/1(din)
  Mult76/1 -> sum/77(din76)
  coeff77/1 -> Mult77/2
  delay76/1(dout) -> Mult77/1, delay77/1(din)
  Mult77/1 -> sum/78(din77)
  coeff78/1 -> Mult78/2
  delay77/1(dout) -> Mult78/1, delay78/1(din)
  Mult78/1 -> sum/79(din78)
  coeff79/1 -> Mult79/2
  delay78/1(dout) -> Mult79/1, delay79/1(din)
  Mult79/1 -> sum/80(din79)
  coeff80/1 -> Mult80/2
  delay79/1(dout) -> Mult80/1, delay80/1(din)
  Mult80/1 -> sum/81(din80)
  coeff81/1 -> Mult81/2
  delay80/1(dout) -> Mult81/1, delay81/1(din)
  Mult81/1 -> sum/82(din81)
  coeff82/1 -> Mult82/2
  delay81/1(dout) -> Mult82/1, delay82/1(din)
  Mult82/1 -> sum/83(din82)
  coeff83/1 -> Mult83/2
  delay82/1(dout) -> Mult83/1, delay83/1(din)
  Mult83/1 -> sum/84(din83)
  coeff84/1 -> Mult84/2
  delay83/1(dout) -> Mult84/1, delay84/1(din)
  Mult84/1 -> sum/85(din84)
  coeff85/1 -> Mult85/2
  delay84/1(dout) -> Mult85/1, delay85/1(din)
  Mult85/1 -> sum/86(din85)
  coeff86/1 -> Mult86/2
  delay85/1(dout) -> Mult86/1, delay86/1(din)
  Mult86/1 -> sum/87(din86)
  coeff87/1 -> Mult87/2
  delay86/1(dout) -> Mult87/1, delay87/1(din)
  Mult87/1 -> sum/88(din87)
  coeff88/1 -> Mult88/2
  delay87/1(dout) -> Mult88/1, delay88/1(din)
  Mult88/1 -> sum/89(din88)
  coeff89/1 -> Mult89/2
  delay88/1(dout) -> Mult89/1, delay89/1(din)
  Mult89/1 -> sum/90(din89)
  coeff90/1 -> Mult90/2
  delay89/1(dout) -> Mult90/1, delay90/1(din)
  Mult90/1 -> sum/91(din90)
  coeff91/1 -> Mult91/2
  delay90/1(dout) -> Mult91/1, delay91/1(din)
  Mult91/1 -> sum/92(din91)
  coeff92/1 -> Mult92/2
  delay91/1(dout) -> Mult92/1, delay92/1(din)
  Mult92/1 -> sum/93(din92)
  coeff93/1 -> Mult93/2
  delay92/1(dout) -> Mult93/1, delay93/1(din)
  Mult93/1 -> sum/94(din93)
  coeff94/1 -> Mult94/2
  delay93/1(dout) -> Mult94/1, delay94/1(din)
  Mult94/1 -> sum/95(din94)
  coeff95/1 -> Mult95/2
  delay94/1(dout) -> Mult95/1, delay95/1(din)
  Mult95/1 -> sum/96(din95)
  coeff96/1 -> Mult96/2
  delay95/1(dout) -> Mult96/1, delay96/1(din)
  Mult96/1 -> sum/97(din96)
  coeff97/1 -> Mult97/2
  delay96/1(dout) -> Mult97/1, delay97/1(din)
  Mult97/1 -> sum/98(din97)
  coeff98/1 -> Mult98/2
  delay97/1(dout) -> Mult98/1, delay98/1(din)
  Mult98/1 -> sum/99(din98)
  coeff99/1 -> Mult99/2
  delay98/1(dout) -> Mult99/1, delay99/1(din)
  Mult99/1 -> sum/100(din99)
  coeff100/1 -> Mult100/2
  delay99/1(dout) -> Mult100/1
  Mult100/1 -> sum/101(din100)
  data_in/1 -> delay1/1(din), Mult1/1
